simulink model "Full_Car"  (R2020b)
settings: Solver=ode23  StartTime=0.0  StopTime=20  RelTol=0.01  AbsTol=0.01
PreLoadFcn:
  | addpath('Functions');
  | addpath('Functions/geometric');
  | addpath('Parameters');
  | vhclParams;
  | Mass = Sprung_mass + Driver_mass + Unsprung_mass_front*2 + Unsprung_mass_rear*2;
  | COG = (Sprung_mass_COG*Sprung_mass + Driver_COG*Driver_mass + Unsprung_mass_COG_front*Unsprung_mass_front*2.*[1 0 1] + Unsprung_mass_COG_rear*Unsprung_mass_rear*2.*[1 0 1])/Mass;
InitFcn:
  | [folder, ~, ~] = fileparts(which (bdroot));
  | cd(folder);
undefined symbols (not defined in the file): aero_CF, aero_CM, aero_pressureCenter, aero_refArea, airDensity, ARB_bcrnk_frontL, ARB_bcrnk_frontR, ARB_bcrnk_rearL, ARB_bcrnk_rearR, ARB_center_front, ARB_center_rear, ARB_damping_front, ARB_damping_rear, ARB_length_front, ARB_length_rear, ARB_pinSlot_correctionFactor_front, ARB_pinSlot_correctionFactor_rear, ARB_stiffness_front, ARB_stiffness_rear, ARB_TieRod_length_front, ARB_TieRod_length_rear, Damper_coefficient_front, Damper_coefficient_rear, Driver_COG, Driver_mass, DriveTrain_brkwyTrq, DriveTrain_brkwyVel, DriveTrain_CoulombTrq, DriveTrain_effcy, DriveTrain_ratio, DriveTrain_viscCoef, Floor_pos, Floor_size, FS_camber, FS_toe, gravity, Grid_size, initialPosition, Rim_mass, RodDiameter, RodThickness, RS_camber, RS_toe, sigbuilder_block, Spring_preload_front, Spring_preload_rear, Spring_stiffness_front, Spring_stiffness_rear, Sprung_mass, Sprung_mass_COG, Tire_mass, Unsprung_mass_COG_front, Unsprung_mass_COG_rear, Unsprung_mass_front, Unsprung_mass_rear, util_generate_grid_x, util_generate_grid_y, util_rodCrossSection, X, X_F ... (+3)
libraries: sm_lib x385, nesl_utility x79, simulink_extras x8, tire x4, fl_ps_legacy_lib x1
(part 1 of 17)
settings Model: Creator=Adri  LastModifiedBy=Adri
settings SolverCC: MaxConsecutiveZCs=10  ZeroCrossControl=DisableAll  ZeroCrossAlgorithm=Adaptive
settings DiagnosticsConfigSet: SimMechanicsUnconnectedFramePorts=none  SimMechanicsJointTargetOverSpecification=warning
settings ExplorerConfigSet: InternalSimMechanicsExplorerSettings="<ExplorerConfiguration><canonicalview>default</canonicalview><AnimationSetting><BasePlaybackSpeed>1.0</BasePlaybackSpeed><PlaybackSpeedFactor>1.0</PlaybackSpeedFactor></AnimationSetting><TilePanel><TileInfo><Tiles Columns=\"2\" Count=\"4\" Rows=\"2\"><Column Weight=\"0.4998\"/><Column Weight=\"0.5002\"/><Row Weight=\"0.4996\"/><Row Weight=\"0.5004\"/><Tile Height=\"1\" Width=\"1\" X=\"0\" Y=\"0\"/><Tile Height=\"1\" Width=\"1\" X=\"0\" Y=\"1\"/><Tile Height=\"1\" Width=\"1\" X=\"1\" Y=\"0\"/><Tile Height=\"1\" Width=\"1\" X=\"1\" Y=\"1\"/></Tiles></TileInfo><SceneInfo><SceneViewer><BGColor><R>0.60000002384185791</R><G>0.60000002384185791</G><B>0.60000002384185791</B></BGColor><Camera><DoPerspective>on</DoPerspective><Position><X>10.0377769209018357</X><Y>-0.000524616729167124785</Y><Z>20.1274277417939516</Z></Position><Center><X>10.2661913871839321</X><Y>-0.000383839117840143265</Y><Z>0.128732114034256717</Z></Center><Up><X>1</X><Y>0</Y><Z>0</Z></Up><ViewLimDist>1</ViewLimDist><YLim>0.466307658154998583</YLim><XLim>0.65312726046828895</XLim></Camera></SceneViewer><SceneViewer><BGColor><R>0.60000002384185791</R><G>0.60000002384185791</G><B>0.60000002384185791</B></BGColor><Camera><DoPerspective>on</DoPerspective><Position><X>13.2317240746009244</X><Y>-3.00041468433522773</Y><Z>3.16277740169981803</Z></Position><Center><X>10.2661913871839321</X><Y>-0.000383839117840143265</Y><Z>0.128732114034256717</Z></Center><Up><X>0</X><Y>0</Y><Z>1</Z></Up><ViewLimDist>1</ViewLimDist><YLim>0.466307658154998583</YLim><XLim>0.651057126520687479</XLim></Camera></SceneViewer><SceneViewer><BGColor><R>0.60000002384185791</R><G>0.60000002384185791</G><B>0.60000002384185791</B></BGColor><Camera><DoPerspective>on</DoPerspective><Position><X>6.23790045540382909</X><Y>-0.000269772856466627211</Y><Z>2.58288617519811803</Z></Position><Center><X>10.2513444468755957</X><Y>-0.000392989662576397069</Y><Z>1.42864732983863707</Z></Center><Up><X>0</X><Y>0</Y><Z>1</Z></Up><ViewLimDist>1</ViewLimDist><YLim>0.466307658154998583</YLim><XLim>0.653868608096516368</XLim></Camera></SceneViewer><SceneViewer><BGColor><R>0.60000002384185791</R><G>0.60000002384185791</G><B>0.60000002384185791</B></BGColor><Camera><DoPerspective>off</DoPerspective><Position><X>111.551441519002438</X><Y>-61.5514434576371485</Y><Z>61.6014420553164683</Z></Position><Center><X>50.0000011746554094</X><Y>-3.11328978241931509e-06</Y><Z>0.0500017109691022199</Z></Center><Up><X>-0.408248290463863073</X><Y>0.408248290463863073</Y><Z>0.816496580927726145</Z></Up><ViewLimDist>1</ViewLimDist><YLim>78.4280247571084601</YLim><XLim>109.625226364135756</XLim></Camera></SceneViewer></SceneInfo></TilePanel><MechEditorPanel><Cameras><Camera><Id>Camera1</Id><Value><Name>Front suspension</Name><NativeCamera><PositionTrackingFeature>Vehicle.Cameras.Front_suspension_pos.F</PositionTrackingFeature><CenterTrackingFeature>Vehicle.Cameras.Front_suspension_aim.F</CenterTrackingFeature><UpVectorTrackingFeature>World_Frame.W</UpVectorTrackingFeature><CenterTrackingOrigin>on</CenterTrackingOrigin><UpVectorTrackingDirection>+Z</UpVectorTrackingDirection></NativeCamera></Value></Camera><Camera><Id>Camera2</Id><Value><Name>Rear Suspension</Name><NativeCamera><PositionTrackingFeature>Vehicle.Cameras.Rear_suspension_pos.F</PositionTrackingFeature><CenterTrackingFeature>Vehicle.Cameras.Rear_suspension_aim.F</CenterTrackingFeature><UpVectorTrackingFeature>World_Frame.W</UpVectorTrackingFeature><CenterTrackingOrigin>on</CenterTrackingOrigin><UpVectorTrackingDirection>+Z</UpVectorTrackingDirection></NativeCamera></Value></Camera><Camera><Id>Camera3</Id><Value><Name>Vehicle isometric</Name><NativeCamera><PositionTrackingFeature>Vehicle.Cameras.Vehicle_Isometric_pos.F</PositionTrackingFeature><CenterTrackingFeature>Vehicle.Cameras.Chassis_Ref</CenterTrackingFeature><UpVectorTrackingFeature>World_Frame.W</UpVectorTrackingFeature><CenterTrackingOrigin>on</CenterTrackingOrigin><UpVectorTrackingDirection>+Z</UpVectorTrackingDirection></NativeCamera></Value></Camera><Camera><Id>Camera4</Id><Value><Name>Vehicle Isometric Fixed</Name><NativeCamera><PositionTrackingFeature>Vehicle.Cameras.Vehicle_Isometric_Fixed_pos.F</PositionTrackingFeature><CenterTrackingFeature>Vehicle.Cameras.Chassis_Ref</CenterTrackingFeature><UpVectorTrackingFeature>World_Frame.W</UpVectorTrackingFeature><CenterTrackingOrigin>on</CenterTrackingOrigin><UpVectorTrackingDirection>+Z</UpVectorTrackingDirection></NativeCamera></Value></Camera><Camera><Id>Camera5</Id><Value><Name>Driver</Name><NativeCamera><PositionTrackingFeature>Vehicle.Cameras.Driver_pos.F</PositionTrackingFeature><CenterTrackingFeature>Vehicle.Cameras.Driver_aim.F</CenterTrackingFeature><UpVectorTrackingFeature>Vehicle.Cameras.Chassis_Ref</UpVectorTrackingFeature><CenterTrackingOrigin>on</CenterTrackingOrigin><UpVectorTrackingDirection>+Z</UpVectorTrackingDirection></NativeCamera></Value></Camera><Camera><Id>Camera6</Id><Value><Name>Forward</Name><NativeCamera><PositionTrackingFeature>Vehicle.Cameras.Forward_pos.F</PositionTrackingFeature><CenterTrackingFeature>Vehicle.Cameras.Forward_aim.F</CenterTrackingFeature><UpVectorTrackingFeature>Vehicle.Cameras.Chassis_Ref</UpVectorTrackingFeature><CenterTrackingOrigin>on</CenterTrackingOrigin><UpVectorTrackingDirection>+Z</UpVectorTrackingDirection></NativeCamera></Value></Camera><Camera><Id>Camera7</Id><Value><Name>Forward high</Name><NativeCamera><PositionTrackingFeature>Vehicle.Cameras.Forward_high_pos.F</PositionTrackingFeature><CenterTrackingFeature>Vehicle.Cameras.Forward_high_aim.F</CenterTrackingFeature><UpVectorTrackingFeature>World_Frame.W</UpVectorTrackingFeature><CenterTrackingOrigin>on</CenterTrackingOrigin><UpVectorTrackingDirection>+Z</UpVectorTrackingDirection></NativeCamera></Value></Camera><Camera><Id>Camera8</Id><Value><Name>Top</Name><NativeCamera><PositionTrackingFeature>Vehicle.Cameras.Top_pos.F</PositionTrackingFeature><CenterTrackingFeature>Vehicle.Cameras.Chassis_Ref</CenterTrackingFeature><UpVectorTrackingFeature>Floor.World</UpVectorTrackingFeature><CenterTrackingOrigin>on</CenterTrackingOrigin><UpVectorTrackingDirection>+X</UpVectorTrackingDirection></NativeCamera></Value></Camera><Camera><Id>Camera9</Id><Value><Name>Front</Name><NativeCamera><PositionTrackingFeature>Vehicle.Cameras.Front.F</PositionTrackingFeature><CenterTrackingFeature>Vehicle.Cameras.Chassis_Ref</CenterTrackingFeature><UpVectorTrackingFeature>Vehicle.Cameras.Chassis_Ref</UpVectorTrackingFeature><CenterTrackingOrigin>on</CenterTrackingOrigin><UpVectorTrackingDirection>+Z</UpVectorTrackingDirection></NativeCamera></Value></Camera><Camera><Id>Camera10</Id><Value><Name>Left</Name><NativeCamera><PositionTrackingFeature>Vehicle.Cameras.Left.F</PositionTrackingFeature><CenterTrackingFeature>Vehicle.Cameras.Left_aim.F</CenterTrackingFeature><UpVectorTrackingFeature>Vehicle.Cameras.Chassis_Ref</UpVectorTrackingFeature><CenterTrackingOrigin>on</CenterTrackingOrigin><UpVectorTrackingDirection>+Z</UpVectorTrackingDirection></NativeCamera></Value></Camera></Cameras><GfxPanelCameraMap><MapElement CameraMLId=\"Camera8\" GfxPanelIndex=\"0\"/><MapElement CameraMLId=\"Camera3\" GfxPanelIndex=\"1\"/><MapElement CameraMLId=\"Camera7\" GfxPanelIndex=\"2\"/></GfxPanelCameraMap><VideoCreator><PlaybackSpeedRatio>1.0</PlaybackSpeedRatio><FrameRate>30</FrameRate><VideoFormat>Motion JPEG AVI</VideoFormat><FrameSize>auto</FrameSize></VideoCreator></MechEditorPanel></ExplorerConfiguration>"

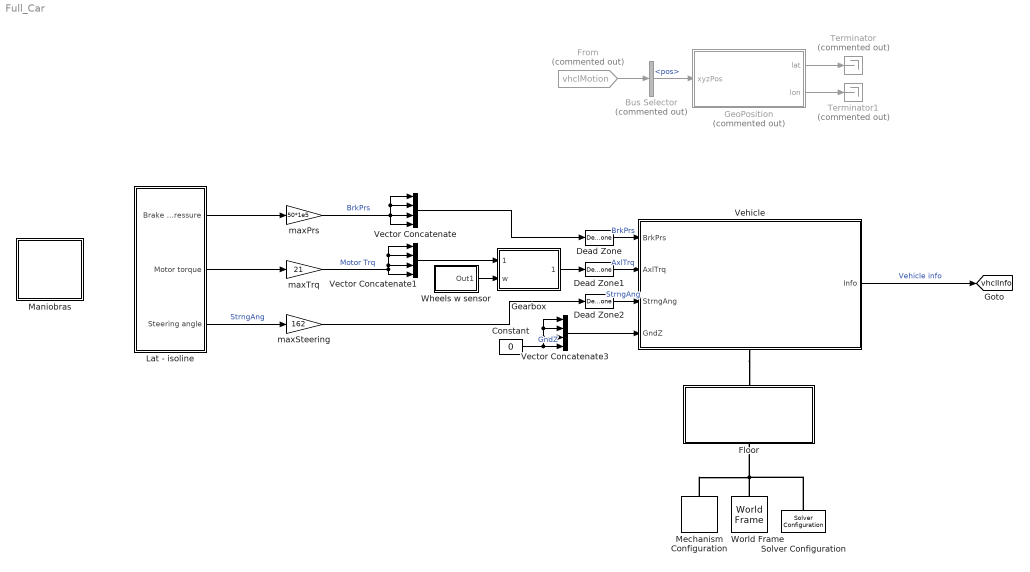
== system Full_Car ==
  Bus Selector : BusSelector  Commented=on  OutputSignals=pos
  Constant : Constant  Value=0
  Dead Zone : DeadZone  LowerValue=-inf  UpperValue=1  ZeroCross=off  SaturateOnIntegerOverflow=on
  Dead Zone1 : DeadZone  LowerValue=-1  UpperValue=1  ZeroCross=off  SaturateOnIntegerOverflow=on
  Dead Zone2 : DeadZone  LowerValue=-1  UpperValue=1  ZeroCross=off  SaturateOnIntegerOverflow=on
  Floor : Subsystem
      settings: PortSchema="{\"entries\":[{\"content\":{\"sides\":[{\"content\":{\"connectorIds\":[]},\"type\":\"ConnectorPlacement.EquallySpacedRectSide\",\"uuid\":\"59915682-4731-433b-9ad1-444682391955\"},{\"content\":{\"connectorIds\":[],\"side\":\"RIGHT\"},\"type\":\"ConnectorPlacement.EquallySpacedRectSide\",\"uuid\":\"de237389-5727-4a62-80fa-d82ee39c04b6\"},{\"content\":{\"connectorIds\":[\"LConn1\"],\"side\":\"TOP\"},\"type\":\"ConnectorPlacement.EquallySpacedRectSide\",\"uuid\":\"42a73084-426d-43e0-98e5-1b64ca34b180\"},{\"content\":{\"connectorIds\":[\"RConn1\"],\"side\":\"BOTTOM\"},\"type\":\"ConnectorPlacement.EquallySpacedRectSide\",\"uuid\":\"3e44a854-086e-4b4c-9c02-9352d24215d0\"}]},\"type\":\"ConnectorPlacement.EquallySpacedConnector\",\"uuid\":\"449c301b-187e-45e2-b186-1f911a16e015\"}],\"packageUris\":[\"http://schema.mathworks.com/mf0/Connector/19700101\"],\"version\":\"1.0\"}"
  From : From  Commented=on  GotoTag=vhclMotion  TagVisibility=scoped
  Gearbox : Subsystem  mask{drvRatio=DriveTrain_ratio, effcy=DriveTrain_effcy, brkwyTrq=DriveTrain_brkwyTrq, brkwyVel=DriveTrain_brkwyVel, CoulombTrq=DriveTrain_CoulombTrq, viscCoef=DriveTrain_viscCoef}
      mask prompts: drvRatio "Drive ratio"; effcy "Efficiency"; brkwyTrq "Breakaway friction torque"; brkwyVel "Breakaway friction velocity"; CoulombTrq "Coulomb friction torque"; viscCoef "Viscous friction coefficient"
      mask DialogControl DescGroupVar Prompt: Drive train
      mask DialogControl drvRatio Tooltip: Transmission ratio between input and output shaft (output/input)
      mask DialogControl effcy Tooltip: Torque transmission efficiency
      mask DialogControl Container3 Prompt: Friction
      mask DialogControl brkwyTrq Tooltip: Breakaway friction torque. Minimum torque on the input to have any torque on the output (Nm)
      mask DialogControl brkwyVel Tooltip: Breakaway friction velocity (rad/s)
      mask DialogControl CoulombTrq Tooltip: Constant friction torque (Nm)
      mask DialogControl viscCoef Tooltip: Viscous friction coefficient (N*m/(rad/s))
  GeoPosition : Subsystem  Commented=on  mask{R=0, refLat=0, refLon=0, refAlt=0, refNorth=0}
      mask prompts: R "Earth radius"; refLat "Latitude"; refLon "Longitude"; refAlt "Altitude"; refNorth "North"
      mask DialogControl DescGroupVar Prompt: GeoPosition
      mask DialogControl DescTextVar Prompt: Calculate geographic coordinates of the vehicle
      mask DialogControl R Tooltip: Earth radius, for the reference latitude and longitude (m)
      mask DialogControl Container3 Prompt: Reference
      mask DialogControl refLat Tooltip: Latitude of the origin (deg)
      mask DialogControl refLon Tooltip: Longitude of the origin (deg)
      mask DialogControl refAlt Tooltip: Altitude of the origin (m)
      mask DialogControl refNorth Tooltip: Degrees of rotation respect to the north direction (deg)
  Lat - isoline : Subsystem  Tag=STV Subsys  LoadFcn=sigbuilder_block('load');  StartFcn=sigbuilder_block('start');  StopFcn=sigbuilder_block('stop');  ClipboardFcn=sigbuilder_block('clipboard');  mask(Sigbuilder block){tuvar=[0:10]}
      mask help: Simulink:masks:SigbuilderBlock_MD
      mask init:
      | if ~strcmp(get_param(bdroot(gcbh),'SimulationStatus'),'stopped') tuvar = sigbuilder_block('maskInit'); end
      settings: CopyFcn=sigbuilder_block('copy');  DeleteFcn=sigbuilder_block('delete');  ModelCloseFcn=sigbuilder_block('modelClose');  PreSaveFcn=sigbuilder_block('preSave');  PauseFcn=sigbuilder_block('pause');  ContinueFcn=sigbuilder_block('continue');  NameChangeFcn=sigbuilder_block('namechange');  OpenFcn=sigbuilder_block('open',[248.25 153 865.95 477 ]);  CloseFcn=sigbuilder_block('close');
      mask Display: plot(0, 0, 100, 100,[2, 2, 32, 32, 2], [68, 8, 8, 68, 68],[32, 2], [38, 38], [32, 19, 2],[53, 60, 44], [32, 17, 17, 2],[16, 16, 31, 31]);txt = getActiveGroup(gcbh);text(2, 100, txt,'verticalAlignment', 'top');
  Maniobras : Subsystem
  Mechanism Configuration : sm_lib/Utilities/Mechanism Configuration  UniformGravity=Constant  GravityVector=[0 0 -gravity]  GravityVectorUnits=m/s^2  GravityUnits=m/s^2  LinearizationDelta=0.001  JointModeTransitionNonlinearIterations=2
  Solver Configuration : nesl_utility/Solver Configuration  PhysicalDomain=network_engine_domain  SubClassName=solver  LeftPortType=input  RightPortType=generic  Tones=[]  Harmonics=[]  SimulateNoise=off  Profile=off  UseLocalSampling=off  EnableSwitchedLinearOptims=on  FrequencyDomain=off  UseCCode=off  RelTol=0.001  AbsTol=1e-06  MinStep=1e-09  ToWorkspace=off  RevertToSquareIcSolve=off  NumHomotopyIterations=100  PhasorMode=off  PhasorModeNumHarmonics=1  DoDC=off  ResidualTolerance=1e-09  UseLocalSolver=off  LocalSolverChoice=NE_BACKWARD_EULER_ADVANCER  LocalSolverSampleTime=0.001  DoFixedCost=off  MaxNonlinIter=3  MaxModeIter=2  ComputeImpulses=off  ImpulseIterations=2  LinearAlgebra=Sparse  EquationFormulation=NE_TIME_EF  DelaysMemoryBudget=1024  AutomaticFiltering=on  FilteringTimeConstant=0.1  PartitionStorageMethod=AS_NEEDED  PartitionMemoryBudget=1024  PartitionMethod=FAST
  Terminator : Terminator  Commented=on
  Terminator1 : Terminator  Commented=on
  Vector Concatenate : Concatenate  NumInputs=4
  Vector Concatenate1 : Concatenate  NumInputs=4
  Vector Concatenate3 : Concatenate  NumInputs=4
  Vehicle : Subsystem
      settings: PortSchema="{\"entries\":[{\"content\":{\"sides\":[{\"content\":{\"connectorIds\":[\"In1\",\"In2\",\"In3\",\"In4\"]},\"type\":\"ConnectorPlacement.EquallySpacedRectSide\",\"uuid\":\"4ba0f89d-2af1-4655-8f71-325e5ad02245\"},{\"content\":{\"connectorIds\":[\"Out1\"],\"side\":\"RIGHT\"},\"type\":\"ConnectorPlacement.EquallySpacedRectSide\",\"uuid\":\"e40e4122-155c-4528-902a-77dc8216dbbe\"},{\"content\":{\"connectorIds\":[],\"side\":\"TOP\"},\"type\":\"ConnectorPlacement.EquallySpacedRectSide\",\"uuid\":\"80d51cd1-2f42-4c41-ac4a-812297993a3c\"},{\"content\":{\"connectorIds\":[\"RConn1\"],\"side\":\"BOTTOM\"},\"type\":\"ConnectorPlacement.EquallySpacedRectSide\",\"uuid\":\"0457f4ce-e2d2-401b-a73a-336035eb9210\"}]},\"type\":\"ConnectorPlacement.EquallySpacedConnector\",\"uuid\":\"a9bd6a3f-05ff-481c-9682-f76d449faaea\"}],\"packageUris\":[\"http://schema.mathworks.com/mf0/Connector/19700101\"],\"version\":\"1.0\"}"
  Wheels w sensor : Subsystem
  World Frame : sm_lib/Frames and Transforms/World Frame
  maxPrs : Gain  Gain=50*1e5
  maxSteering : Gain  Gain=162
  maxTrq : Gain  Gain=21
  tag scope: vhclInfo=global
  wires:
  Lat - isoline/1(Brake pressure) -> maxPrs/1
  Lat - isoline/3(Steering angle) -> maxSteering/1  "StrngAng"
  Dead Zone/1 -> Vehicle/1(BrkPrs)  "BrkPrs"
  Dead Zone1/1 -> Vehicle/2(AxlTrq)  "AxlTrq"
  Dead Zone2/1 -> Vehicle/3(StrngAng)  "StrngAng"
  Vehicle/1(Info) -> [vhclInfo]  "Vehicle info"
  GeoPosition/1(lat) -> Terminator/1
  GeoPosition/2(lon) -> Terminator1/1
  From/1 -> Bus Selector/1
  Bus Selector/1 -> GeoPosition/1(xyzPos)  "<pos>"
  Lat - isoline/2(Motor torque) -> maxTrq/1
  Vector Concatenate/1 -> Dead Zone/1
  maxPrs/1 -> Vector Concatenate/3, Vector Concatenate/4, Vector Concatenate/1, Vector Concatenate/2  "BrkPrs"
  Constant/1 -> Vector Concatenate3/2, Vector Concatenate3/1, Vector Concatenate3/3, Vector Concatenate3/4  "GndZ"
  Vector Concatenate3/1 -> Vehicle/4(GndZ)
  maxSteering/1 -> Dead Zone2/1
  Vector Concatenate1/1 -> Gearbox/1(Motor Trq)
  Gearbox/1(AxlTrq) -> Dead Zone1/1
  Wheels w sensor/1(Out1) -> Gearbox/2(w)
  maxTrq/1 -> Vector Concatenate1/4, Vector Concatenate1/3, Vector Concatenate1/1, Vector Concatenate1/2  "Motor Trq"
  connections:
  World Frame/RConn1 -- Mechanism Configuration/RConn1 -- Solver Configuration/RConn1 -- Floor/RConn1
  Vehicle/RConn1 -- Floor/LConn1

== system Full_Car/Floor ==
  Brick Solid : sm_lib/Body Elements/Brick Solid  GraphicAmbientColor=[0.15 0.15 0.15 1.0]  BasedOnType=Density  BrickDimensions=Floor_size  BrickDimensionUnits=m  CenterOfMass=[0 0 0]  CenterOfMassUnits=m  GraphicDiffuseColor=[0.5 0.5 0.5]  Density=1000  DensityUnits=kg/m^3  DoExposeReferenceFrame=on  GraphicEmissiveColor=[0.0 0.0 0.0 1.0]  ExportEntireGeometry=off  SerializedFrames=<Frames/>  MarkerShape=Sphere  MarkerSize=10  GraphicType=FromGeometry  InertiaType=CalculateFromGeometry  Mass=1  MassUnits=kg  MomentsOfInertia=[1 1 1]  MomentsOfInertiaUnits=kg*m^2  GraphicOpacity=1.0  ProductsOfInertia=[0 0 0]  ProductsOfInertiaUnits=kg*m^2  GraphicShininess=75  GraphicSpecularColor=[0.5 0.5 0.5 1.0]  GraphicVisPropType=SimpleVisualProperties
  Grid x : sm_lib/Curves and Surfaces/Spline  GraphicAmbientColor=[0.15 0.15 0.15 1.0]  GraphicDiffuseColor=[0.0 0.0 0.0]  DataPoints=util_generate_grid_x(Floor_size(1),Grid_size)  DataPointsUnits=m  GraphicEmissiveColor=[0.0 0.0 0.0 1.0]  EndConditionType=Natural  MarkerShape=Sphere  MarkerSize=10  GraphicType=FromGeometry  GraphicOpacity=1.0  GraphicShininess=75  GraphicSpecularColor=[0.5 0.5 0.5 1.0]  GraphicVisPropType=SimpleVisualProperties
  Grid y : sm_lib/Curves and Surfaces/Spline  GraphicAmbientColor=[0.15 0.15 0.15 1.0]  GraphicDiffuseColor=[0.0 0.0 0.0]  DataPoints=util_generate_grid_y(Floor_size(2),Grid_size)  DataPointsUnits=m  GraphicEmissiveColor=[0.0 0.0 0.0 1.0]  EndConditionType=Natural  MarkerShape=Sphere  MarkerSize=10  GraphicType=FromGeometry  GraphicOpacity=1.0  GraphicShininess=75  GraphicSpecularColor=[0.5 0.5 0.5 1.0]  GraphicVisPropType=SimpleVisualProperties
  Rigid Transform : sm_lib/Frames and Transforms/Rigid Transform  TranslationMethod=StandardAxis  TranslationStandardOffset=Floor_size(3)/2  TranslationLengthUnit=m  TranslationStandardAxis=+Z  TranslationCartesianOffset=[0 0 0]  TranslationROffset=0  TranslationROffsetUnits=m  TranslationZOffset=0  TranslationZOffsetUnits=m  TranslationThetaOffset=0  TranslationThetaOffsetUnits=deg  RotationMethod=None  RotationAngleUnits=deg  RotationStandardAxis=+Z  RotationAngle=0.0  RotationArbitraryAxis=[0 0 1]  FollAlignAxisA=+X  BaseAlignAxisA=+Y  FollAlignAxisB=+Y  BaseAlignAxisB=+Z  RotationSequenceAxes=FollowerAxes  RotationSequence=XYX  RotationSequenceAngles=[0 0 0]  RotationSequenceAnglesUnits=deg  RotationMatrix=[1 0 0; 0 1 0; 0 0 1]
  Rigid Transform1 : sm_lib/Frames and Transforms/Rigid Transform  TranslationMethod=Cartesian  TranslationStandardOffset=0  TranslationLengthUnit=m  TranslationStandardAxis=+Z  TranslationCartesianOffset=[Floor_pos(1) Floor_pos(2) Floor_pos(3)]  TranslationROffset=0  TranslationROffsetUnits=m  TranslationZOffset=0  TranslationZOffsetUnits=m  TranslationThetaOffset=0  TranslationThetaOffsetUnits=deg  RotationMethod=None  RotationAngleUnits=deg  RotationStandardAxis=+Z  RotationAngle=0.0  RotationArbitraryAxis=[0 0 1]  FollAlignAxisA=+X  BaseAlignAxisA=+Y  FollAlignAxisB=+Y  BaseAlignAxisB=+Z  RotationSequenceAxes=FollowerAxes  RotationSequence=XYX  RotationSequenceAngles=[0 0 0]  RotationSequenceAnglesUnits=deg  RotationMatrix=[1 0 0; 0 1 0; 0 0 1]
  Ref : PMIOPort  Side=Left
  World : PMIOPort  Port=2  Side=Right
  connections:
  Ref/RConn1 -- Rigid Transform/RConn1 -- Grid x/LConn1 -- Grid y/LConn1
  Rigid Transform1/RConn1 -- Rigid Transform/LConn1 -- Brick Solid/RConn1
  Rigid Transform1/LConn1 -- World/RConn1

== system Full_Car/Gearbox ==
  Motor Trq : Inport
  w : Inport  Port=2
  Drive ratio : Gain  Gain=drvRatio
  Efficiency : Gain  Gain=effcy
  Rotational friction : Subsystem  mask{brkwyTrq=brkwyTrq, brkwyVel=brkwyVel, CoulombTrq=CoulombTrq, viscCoef=viscCoef}
      mask prompts: brkwyTrq "Breakaway friction torque"; brkwyVel "Brakaway friction velocity"; CoulombTrq "Coulomb friction torque"; viscCoef "Viscous friction coefficient"
      mask DialogControl ParameterGroupVar Prompt: Rotational friction
  AxlTrq : Outport
  wires:
  Motor Trq/1 -> Drive ratio/1
  Drive ratio/1 -> Efficiency/1
  Efficiency/1 -> Rotational friction/1(inTrq)
  w/1 -> Rotational friction/2(w)
  Rotational friction/1(outTrq) -> AxlTrq/1

== system Full_Car/GeoPosition ==
  xyzPos : Inport
  Constant : Constant  Value=refNorth
  Degrees to Radians : simulink_extras/Transformations/Degrees to Radians
  Initial heading correction : MATLAB Function  TreatAsAtomicUnit=on
  cart2Geo : Subsystem
  lat : Outport
  lon : Outport  Port=2
  wires:
  xyzPos/1 -> Initial heading correction/2(u)
  Initial heading correction/1(y) -> cart2Geo/1(<pos>)
  Degrees to Radians/1 -> Initial heading correction/1(yaw0)
  Constant/1 -> Degrees to Radians/1
  cart2Geo/1(Lat) -> lat/1  "Latitude"
  cart2Geo/2(Lon) -> lon/1  "Longitude"

== system Full_Car/Lat - isoline ==
  Demux : Demux  Tag=STV Demux  Outputs=3
      settings: DisplayOption=none
  FromWs : FromWorkspace  Tag=STV FromWs  VariableName=tuvar
      SigBuilderData=|
      | common.afterFinalStr = 'Extrapolate'
      | common.sampleTime = 0
      | dataSet.timeRange = [0 100]
      | dataSet.name = 'Input signals'
      | sbobj.Groups.Name = 'Input signals'
      | sbobj.Groups.Signals(1).Name = 'Brake pressure'
      | sbobj.Groups.Signals(1).XData = [0 92.3 92.3 93.5 93.5 100]
      | sbobj.Groups.Signals(1).YData = [0 0 1.21 1.21 0 0]
      | sbobj.Groups.Signals(2).Name = 'Motor torque'
      | sbobj.Groups.Signals(2).XData = [0 1.1 2.7 5 7.6 8.7 20 20 93.1 93.1 94.9 94.9 100]
      | sbobj.Groups.Signals(2).YData = [0 0.34 0.46 0.15 0.1365 0.19 0.19 -0.000770550857284 -0.00911651728553 1.2 1.2 -0.00934699103713 -0.01]
      | sbobj.Groups.Signals(3).Name = 'Steering angle'
      | sbobj.Groups.Signals(3).XData = [0 3 3.2 3.5 4.1 4.3 4.58 7.5 8.7 20 20 87.6 87.6 92.8 92.8 94.6 94.6 100]
      | sbobj.Groups.Signals(3).YData = [0 0 0.3 0.3 -0.3 -0.3 0 0 -0.14 -0.14 0 0 -0.68 -0.68 1.17 1.17 0 0]
      | sbobj.Groups.TimeRange = [0 100]
  Brake pressure : Outport  Tag=STV Outport
  Motor torque : Outport  Tag=STV Outport  Port=2
  Steering angle : Outport  Tag=STV Outport  Port=3
  wires:
  FromWs/1 -> Demux/1
  Demux/1 -> Brake pressure/1
  Demux/2 -> Motor torque/1
  Demux/3 -> Steering angle/1

== system Full_Car/Maniobras ==
  Acc+Brk : Subsystem  Tag=STV Subsys  LoadFcn=sigbuilder_block('load');  StartFcn=sigbuilder_block('start');  StopFcn=sigbuilder_block('stop');  ClipboardFcn=sigbuilder_block('clipboard');  mask(Sigbuilder block){tuvar=[0:10]}
      mask help: Simulink:masks:SigbuilderBlock_MD
      mask init:
      | if ~strcmp(get_param(bdroot(gcbh),'SimulationStatus'),'stopped') tuvar = sigbuilder_block('maskInit'); end
      settings: CopyFcn=sigbuilder_block('copy');  DeleteFcn=sigbuilder_block('delete');  ModelCloseFcn=sigbuilder_block('modelClose');  PreSaveFcn=sigbuilder_block('preSave');  PauseFcn=sigbuilder_block('pause');  ContinueFcn=sigbuilder_block('continue');  NameChangeFcn=sigbuilder_block('namechange');  OpenFcn=sigbuilder_block('open',[591 244.5 860.25 552.75 ]);  CloseFcn=sigbuilder_block('close');
      mask Display: plot(0, 0, 100, 100,[2, 2, 32, 32, 2], [68, 8, 8, 68, 68],[32, 2], [38, 38], [32, 19, 2],[53, 60, 44], [32, 17, 17, 2],[16, 16, 31, 31]);txt = getActiveGroup(gcbh);text(2, 100, txt,'verticalAlignment', 'top');
  Lat : Subsystem  Tag=STV Subsys  LoadFcn=sigbuilder_block('load');  StartFcn=sigbuilder_block('start');  StopFcn=sigbuilder_block('stop');  ClipboardFcn=sigbuilder_block('clipboard');  mask(Sigbuilder block){tuvar=[0:10]}
      mask help: Simulink:masks:SigbuilderBlock_MD
      mask init:
      | if ~strcmp(get_param(bdroot(gcbh),'SimulationStatus'),'stopped') tuvar = sigbuilder_block('maskInit'); end
      settings: CopyFcn=sigbuilder_block('copy');  DeleteFcn=sigbuilder_block('delete');  ModelCloseFcn=sigbuilder_block('modelClose');  PreSaveFcn=sigbuilder_block('preSave');  PauseFcn=sigbuilder_block('pause');  ContinueFcn=sigbuilder_block('continue');  NameChangeFcn=sigbuilder_block('namechange');  OpenFcn=sigbuilder_block('open',[2538.75 -38.25 860.25 552.75 ]);  CloseFcn=sigbuilder_block('close');
      mask Display: plot(0, 0, 100, 100,[2, 2, 32, 32, 2], [68, 8, 8, 68, 68],[32, 2], [38, 38], [32, 19, 2],[53, 60, 44], [32, 17, 17, 2],[16, 16, 31, 31]);txt = getActiveGroup(gcbh);text(2, 100, txt,'verticalAlignment', 'top');
  Lat - isoline : Subsystem  Tag=STV Subsys  LoadFcn=sigbuilder_block('load');  StartFcn=sigbuilder_block('start');  StopFcn=sigbuilder_block('stop');  ClipboardFcn=sigbuilder_block('clipboard');  mask(Sigbuilder block){tuvar=[0:10]}
      mask help: Simulink:masks:SigbuilderBlock_MD
      mask init:
      | if ~strcmp(get_param(bdroot(gcbh),'SimulationStatus'),'stopped') tuvar = sigbuilder_block('maskInit'); end
      settings: CopyFcn=sigbuilder_block('copy');  DeleteFcn=sigbuilder_block('delete');  ModelCloseFcn=sigbuilder_block('modelClose');  PreSaveFcn=sigbuilder_block('preSave');  PauseFcn=sigbuilder_block('pause');  ContinueFcn=sigbuilder_block('continue');  NameChangeFcn=sigbuilder_block('namechange');  OpenFcn=sigbuilder_block('open',[248.25 153 865.95 477 ]);  CloseFcn=sigbuilder_block('close');
      mask Display: plot(0, 0, 100, 100,[2, 2, 32, 32, 2], [68, 8, 8, 68, 68],[32, 2], [38, 38], [32, 19, 2],[53, 60, 44], [32, 17, 17, 2],[16, 16, 31, 31]);txt = getActiveGroup(gcbh);text(2, 100, txt,'verticalAlignment', 'top');
